simulink model "HvacEvaprTFilMgr"  (R2022b)
settings: Solver=FixedStepDiscrete  StartTime=0.0  StopTime=10.0  FixedStep=1  RelTol=1e-3
settings Model: Creator=blue.ge  LastModifiedBy=blue.ge
settings DataIOCC: SaveFormat=StructureWithTime
settings OptimizationCC: DefaultParameterBehavior=Inlined  EnhancedBackFolding=on  PassReuseOutputArgsAs=Individual arguments  ZeroExternalMemoryAtStartup=off  ZeroInternalMemoryAtStartup=off  OptimizeBlockOrder=speed  DifferentSizesBufferReuse=on  OptimizationCustomize=off  EfficientTunableParamExpr=on
settings DebuggingCC: ParameterTunabilityLossMsg=error
settings ModelReferenceCC: ModelReferenceNumInstancesAllowed=Single
settings SFSimCC: MATLABDynamicMemAlloc=off
settings RTWCC: Description=AUTOSAR  SystemTargetFile=autosar.tlc  GenCodeOnly=on  TemplateMakefile=ert_default_tmf
settings CodeAppCC: ForceParamTrailComments=on  IgnoreCustomStorageClasses=off  OperatorAnnotations=on  SimulinkDataObjDesc=on  SFDataObjDesc=on  InsertBlockDesc=on
settings STFCustomTargetCC: TargetLangStandard=C99 (ISO)  MultiwordLength=256  ParMdlRefBuildCompliant=on  CompOptLevelCompliant=on  IncludeMdlTerminateFcn=off  CombineOutputUpdateFcns=on  SuppressErrorStatus=on  ERTCustomFileBanners=on  SupportNonFinite=off  SupportComplex=off  SupportContinuousTime=off  SupportNonInlinedSFcns=off  RemoveResetFunc=on  MATLABClassNameForMDSCustomization=Simulink.SoftwareTarget.ERTCustomization  AutosarCompliant=on  UseToolchainInfoCompliant=on  LookupTableObjectStructAxisOrder=2,1,3,4,...  LUTObjectStructOrderEvenSpacing=Size,Table,Breakpoints  SystemTargetFile=autosar.tlc
settings ERTTargetCC: TargetLangStandard=C99 (ISO)  ConcurrentExecutionCompliant=off  IncludeMdlTerminateFcn=off  SuppressErrorStatus=on  SupportNonFinite=off  SupportComplex=off  ModelStepFunctionPrototypeControlCompliant=off  CPPClassGenCompliant=off  AutosarCompliant=on  LookupTableObjectStructAxisOrder=2,1,3,4,...  LUTObjectStructOrderEvenSpacing=Size,Table,Breakpoints  InlinedParameterPlacement=NonHierarchical  ERTCustomFileTemplate=example_file_process.tlc

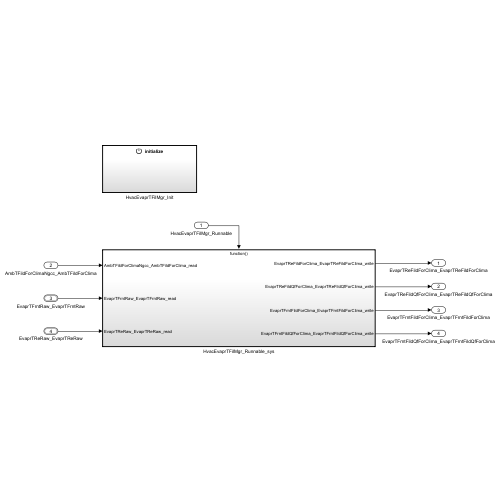
[thumbnail: Simulink's stored preview of the top-level diagram]
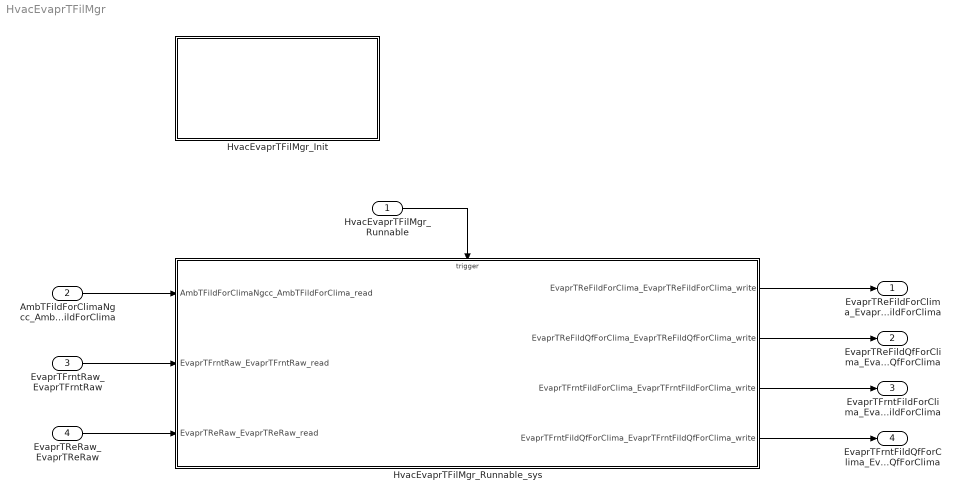
== system HvacEvaprTFilMgr ==
  HvacEvaprTFilMgr_Runnable : Inport  SampleTime=1  OutputFunctionCall=on
  AmbTFildForClimaNgcc_AmbTFildForClima : Inport  Port=2  OutDataTypeStr=T30  PortDimensions=1
  EvaprTFrntRaw_EvaprTFrntRaw : Inport  Port=3  OutDataTypeStr=Bus: EvaprTFrnt  PortDimensions=1  BusOutputAsStruct=on
  EvaprTReRaw_EvaprTReRaw : Inport  Port=4  OutDataTypeStr=Bus: EvaprTFrnt  PortDimensions=1  BusOutputAsStruct=on
  HvacEvaprTFilMgr_Init : Subsystem
  HvacEvaprTFilMgr_Runnable_sys : Subsystem
  EvaprTReFildForClima_EvaprTReFildForClima : Outport  OutDataTypeStr=T30  PortDimensions=1
  EvaprTReFildQfForClima_EvaprTReFildQfForClima : Outport  Port=2  OutDataTypeStr=Boolean  PortDimensions=1
  EvaprTFrntFildForClima_EvaprTFrntFildForClima : Outport  Port=3  OutDataTypeStr=T30  PortDimensions=1
  EvaprTFrntFildQfForClima_EvaprTFrntFildQfForClima : Outport  Port=4  OutDataTypeStr=Boolean  PortDimensions=1
  wires:
  AmbTFildForClimaNgcc_AmbTFildForClima/1 -> HvacEvaprTFilMgr_Runnable_sys/1(AmbTFildForClimaNgcc_AmbTFildForClima_read)
  EvaprTFrntRaw_EvaprTFrntRaw/1 -> HvacEvaprTFilMgr_Runnable_sys/2(EvaprTFrntRaw_EvaprTFrntRaw_read)
  EvaprTReRaw_EvaprTReRaw/1 -> HvacEvaprTFilMgr_Runnable_sys/3(EvaprTReRaw_EvaprTReRaw_read)
  HvacEvaprTFilMgr_Runnable_sys/1(EvaprTReFildForClima_EvaprTReFildForClima_write) -> EvaprTReFildForClima_EvaprTReFildForClima/1
  HvacEvaprTFilMgr_Runnable_sys/2(EvaprTReFildQfForClima_EvaprTReFildQfForClima_write) -> EvaprTReFildQfForClima_EvaprTReFildQfForClima/1
  HvacEvaprTFilMgr_Runnable_sys/3(EvaprTFrntFildForClima_EvaprTFrntFildForClima_write) -> EvaprTFrntFildForClima_EvaprTFrntFildForClima/1
  HvacEvaprTFilMgr_Runnable_sys/4(EvaprTFrntFildQfForClima_EvaprTFrntFildQfForClima_write) -> EvaprTFrntFildQfForClima_EvaprTFrntFildQfForClima/1
  HvacEvaprTFilMgr_Runnable/1 -> HvacEvaprTFilMgr_Runnable_sys/Trigger

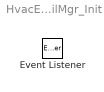
== system HvacEvaprTFilMgr/HvacEvaprTFilMgr_Init ==
  Event Listener : EventListener  EventName=reset

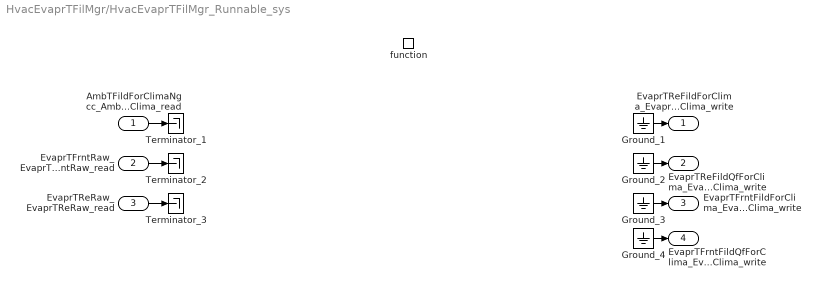
== system HvacEvaprTFilMgr/HvacEvaprTFilMgr_Runnable_sys ==
  AmbTFildForClimaNgcc_AmbTFildForClima_read : Inport
  EvaprTFrntRaw_EvaprTFrntRaw_read : Inport  Port=2
  EvaprTReRaw_EvaprTReRaw_read : Inport  Port=3
  function : TriggerPort  TriggerType=function-call  VariantControl=(inherit)  SampleTimeType=periodic  SampleTime=-1
  Ground_{1..4} (4 alike) : Ground
  Terminator_1 : Terminator
  Terminator_2 : Terminator
  Terminator_3 : Terminator
  EvaprTReFildForClima_EvaprTReFildForClima_write : Outport
      settings: EnsureOutportIsVirtual=on
  EvaprTReFildQfForClima_EvaprTReFildQfForClima_write : Outport  Port=2
      settings: EnsureOutportIsVirtual=on
  EvaprTFrntFildForClima_EvaprTFrntFildForClima_write : Outport  Port=3
      settings: EnsureOutportIsVirtual=on
  EvaprTFrntFildQfForClima_EvaprTFrntFildQfForClima_write : Outport  Port=4
      settings: EnsureOutportIsVirtual=on
  wires:
  AmbTFildForClimaNgcc_AmbTFildForClima_read/1 -> Terminator_1/1
  EvaprTFrntRaw_EvaprTFrntRaw_read/1 -> Terminator_2/1
  EvaprTReRaw_EvaprTReRaw_read/1 -> Terminator_3/1
  Ground_1/1 -> EvaprTReFildForClima_EvaprTReFildForClima_write/1
  Ground_2/1 -> EvaprTReFildQfForClima_EvaprTReFildQfForClima_write/1
  Ground_3/1 -> EvaprTFrntFildForClima_EvaprTFrntFildForClima_write/1
  Ground_4/1 -> EvaprTFrntFildQfForClima_EvaprTFrntFildQfForClima_write/1
